simulink model "TosAeb"  (R2021a)
settings: Solver=FixedStepDiscrete  StartTime=0.0  StopTime=inf  FixedStep=0.01  RelTol=1e-3
undefined symbols (not defined in the file or in its folder): ACCModSts, AccSts1, AEB_ObjectMovingOrientation, AEB_ObjectMovingState, CN_BTCActive, CN_CurrentGearR, CN_CurrentGearR_DHT, CN_PTCActive, CN_VDCActive, CN_VSC_EspOff, CN_VSC_FCW_AEB_Error, CN_VSC_FCW_AEB_NoDispay, CN_VSC_FCW_AEB_PerfmDgdtn, CN_VSC_FCW_AEB_TempUnAvlbl, CN_VSC_VehStandstill, CNaccelAvoidMax, CNaccelAvoidMin, CNbrakePedalPress, CNcomfortStrLeftAccelMax, CNcomfortStrLeftAccelMin, CNcomfortStrRightAccelMax, CNcomfortStrRightAccelMin, CNDrvDoorOpen, CNDrvModeEco, CNDrvModeNorm, CNDrvModeOther, CNDrvModeSport, CNDrvSBRreminder, CNNull, CNobjInvalidID, CNPassDoorOpen, CNPassSBRreminder, CNPEBSAccelNegInf, CNPEBSAccelNull, CNPEBSTimeInf, CNPEBSTimeNull, CNSTOPMAX, CNSTOPNULL, CNsubjWidth, CNSwtOFF, CNSwtON, CNTTCMAX, CNTTCNULL, CNVehConfigAEBCycNone, CNVehConfigAEBNone, CNVehConfigAEBPedNone, CNVehConfigFCWNone, CNVehConfigJANone, CollisionSeverity, FALSE, FCWSnvty, JACycObjCalcInfoDefaultParams, JAPedObjCalcInfoDefaultParams, JAVehObjCalcInfoDefaultParams, latCycObjCalcInfoDefaultParams, latPedObjCalcInfoDefaultParams, longObjCalcInfoDefaultParams, ModSts, movingStatus, ObjectType ... (+1112)
libraries: simulink x3031, AEBSFunctionLib x50, Ta_Library x33, AEBSStateMachineLib x23, Mylibrary x12, simulink_extras x7, ObjSelect_lib x3, dspstat3 x2
(part 92 of 102)

== stateflow chart TosAeb/FcwAebJa_Step_sys/ALG_AEBSystem/ALG_AEBController/AEB_JaObjCalcApp/JaCycTgtInfoCalc/TargetSelectCalc/tgtSelectFlow/tgtObjFlow ==
  state TargetSelectFunc [function]
    note: change dy to dx
    (default) -> (junction 63) : {
        | 	tempObjPreSelectCond = ObjPreSelectCondFufilled(index);
        | 	tempFlg = JaCycObjCalcInfo(index).JaCycObjColliInfo.ObjColliFlg;
        | }
    (junction 63) -> (junction 75) [order 1] : [tempObjPreSelectCond== true &&...
        | tempFlg == true &&...
        | abs(SteerWheelAngle_Deg) > P_JaCycCA_SelectStrWheelAngleAbsThres &&...
        | subjVel <= P_JaCycCA_SelectSubjVelThres]
    (junction 63) -> (junction 69) [order 2]
    (junction 75) -> (junction 311)
    (junction 311) -> (junction 312) [order 1] : [ObjPreSelectCondFufilled(index) == false]
    (junction 311) -> (junction 318) [order 2]
    (junction 312) -> (junction 315)
    (junction 318) -> (junction 321) [order 1] : [ObjPreSelectCondFufilled(tgtIndex) == false ||...
        | JaCycObjCalcInfo(index).JaCycObjColliInfo.ObjColliFlg == false]
    (junction 321) -> (junction 323) : {
        | 	tgtIndex = index;
        | }
    (junction 318) -> (junction 128) [order 2] : {
        | 	tgtIsFind = true;
        | 	temp1dx = abs(JaCycObjCalcInfo(index).JaCycObj.dx);
        | 	temp2dx = abs(JaCycObjCalcInfo(tgtIndex).JaCycObj.dx);
        | 	temp1t = JaCycObjCalcInfo(index).JaCycObjColliInfo.tPredictTime;
        | 	temp2t = JaCycObjCalcInfo(tgtIndex).JaCycObjColliInfo.tPredictTime;
        | }
    (junction 128) -> (junction 131) [order 1] : [temp1t < temp2t]
    (junction 128) -> (junction 292) [order 2] : {
        | 	temp = abs(temp1t - temp2t);
        | }
    (junction 131) -> (junction 289) : {tgtIndex = index;}
    (junction 292) -> (junction 294) [order 1] : [temp < 1e-6&&...
        |  temp1dx < temp2dx]
    (junction 292) -> (junction 101) [order 2]
    (junction 294) -> (junction 296) : {tgtIndex = index;}
    (junction 101) -> (junction 69)
    (junction 296) -> (junction 101)
    (junction 289) -> (junction 296)
    (junction 323) -> (junction 289)
    (junction 315) -> (junction 323)
    (junction 69) -> (junction 81)
  (default) -> (junction 111) : {
      | 	tgtIsFind = false;
      | }
  (junction 111) -> (junction 201) [order 1] : [TargetIDK1 ~=  CNobjInvalidID]
  (junction 111) -> (junction 286) [order 2]
  (junction 201) -> (junction 236)
  (junction 236) -> (junction 238) [order 1] : [JACycPrefillModSts == ModSts.ActiveState ||...
      | JACycAEBfullModSts == ModSts.ActiveState ]
  (junction 236) -> (junction 284) [order 2]
  (junction 238) -> (junction 282) : {
      | 	tgtIndex = tgtIndexK1;
      | 	tgtIsFind = true;
      | }
  (junction 284) -> (junction 286)
  (junction 286) -> (junction 112) : {
      | 	tgtIndex = uint8(1);
      | 	index = uint8(1);
      | }
  (junction 112) -> (junction 110) [order 1] : [index <= 32]
  (junction 113) -> (junction 112) : {index = index + 1;}
  (junction 112) -> (junction 114) [order 2]
  (junction 110) -> (junction 113) : {
      | 	TargetSelectFunc();
      | }
  (junction 282) -> (junction 114)
  (junction 114) -> (junction 220)
  (junction 220) -> (junction 222) [order 1] : [tgtIsFind == true]
  (junction 220) -> (junction 223) [order 2] : {
      | 	JaCycTgtObjInfo = JACycObjCalcInfoDefaultParams;
      | }
  (junction 222) -> (junction 221) : {
      | 	JaCycTgtObjInfo = JaCycObjCalcInfo(tgtIndex);
      | }
  (junction 221) -> (junction 223)
  (junction 223) -> (junction 224)
  data: JaCycObjCalcInfo input Bus: BusAEBJaCycObjCalcInfo size=32; index local uint8; JaCycTgtObjInfo output Bus: BusAEBJaCycObjCalcInfo; temp2t local single; temp1t local single; ObjPreSelectCondFufilled input boolean size=32; subjVel input single; SteerWheelAngle_Deg input single; JACycAEBfullModSts input Enum: ModSts; JACycPrefillModSts input Enum: ModSts; TargetIDK1 input uint16; tgtIndex output uint8; tgtIndexK1 input uint8; tgtIsFind local; JACycObjCalcInfoDefaultParams parameter Bus: BusAEBJaCycObjCalcInfo; CNobjInvalidID parameter single; temp1dx local single; temp2dx local single; tempFlg local; tempObjPreSelectCond local boolean; P_JaCycCA_SelectStrWheelAngleAbsThres input single; P_JaCycCA_SelectSubjVelThres input single

== stateflow chart TosAeb/FcwAebJa_Step_sys/ALG_AEBSystem/ALG_AEBController/AEB_JaObjCalcApp/JaCycTgtInfoCalc/JaCycObjCalcInfoCalc/JaCycObjInfoCalc/ObjCrossFlgCal/CollisionProbCalc/Subsystem/ObjLenthWidthCalc/ObjLengthWidthCalc1 ==
  (default) -> (junction 32)
  (junction 32) -> (junction 34) [order 1] : [ObjectTypeCameraConfirmed == true]
  (junction 32) -> (junction 30) [order 2] : {
      | 	ObjectLength = P_LatPedCA_CollisionRiskProbLengthThres;
      | 	ObjectWidth = P_LatPedCA_CollisionRiskProbWidthThres;
      | }
  (junction 34) -> (junction 36) : {
      | 	ObjectLength = ObjectLengthIn;
      | 	ObjectWidth = ObjectWidthIn;
      | }
  (junction 36) -> (junction 30)
  (junction 30) -> (junction 10)
  (junction 10) -> (junction 12) [order 1] : [(abs(ObjectYawAngle) > 45 &&...
      | abs(ObjectYawAngle) < 135)||...
      | ObjectMovingOrientation == AEB_ObjectMovingOrientation.Crossing]
  (junction 10) -> (junction 16) [order 2]
  (junction 12) -> (junction 14) : {
      |       temp = ObjectLength;
      | 	ObjectLength = ObjectWidth;
      | 	ObjectWidth = temp;
      | }
  (junction 14) -> (junction 16)
  (junction 16) -> (junction 19) : {
      |    ObjectLength = min(ObjectLength,2);
      |    ObjectWidth = min(ObjectWidth,1);
      | }
  data: ObjectYawAngle input; ObjectTypeCameraConfirmed input boolean; ObjectLengthIn input; ObjectWidthIn input; ObjectMovingOrientation input; ObjectLength output single; ObjectWidth output single; P_LatPedCA_CollisionRiskProbLengthThres input; P_LatPedCA_CollisionRiskProbWidthThres input; temp local single

== stateflow chart TosAeb/FcwAebJa_Step_sys/ALG_AEBSystem/ALG_AEBInput/LongObjInfoMap/Subsystem/ObjType_Subsystem/ObjectType ==
  (default) -> (junction 17)
  (junction 17) -> (junction 18) [order 1] : [ObjCar==1]
  (junction 17) -> (junction 19) [order 2]
  (junction 18) -> (junction 23) : {ObjType = 2;}
  (junction 19) -> (junction 20) [order 1] : [ObjTruck ==1]
  (junction 19) -> (junction 26) [order 2]
  (junction 20) -> (junction 22) : {
      | 	ObjType=3;
      | }
  (junction 26) -> (junction 27) [order 1] : [ObjPed==1]
  (junction 26) -> (junction 83) [order 2]
  (junction 27) -> (junction 29) : {
      | 	ObjType=6;
      | }
  (junction 83) -> (junction 85) [order 1] : [ObjMotor==1]
  (junction 83) -> (junction 91) [order 2]
  (junction 85) -> (junction 87) : {ObjType=4;}
  (junction 91) -> (junction 93) [order 1] : [ObjBicycle==1]
  (junction 91) -> (junction 100) [order 2]
  (junction 93) -> (junction 95) : {ObjType=5;}
  (junction 100) -> (junction 102) [order 1] : [ObjGeneral==1]
  (junction 100) -> (junction 21) [order 2] : {ObjType=1;}
  (junction 102) -> (junction 104) : {ObjType=7;}
  (junction 104) -> (junction 21)
  (junction 95) -> (junction 104)
  (junction 87) -> (junction 95)
  (junction 29) -> (junction 87)
  (junction 22) -> (junction 29)
  (junction 23) -> (junction 22)
  (junction 21) -> (junction 25)
  data: ObjCar input; ObjTruck input; ObjPed input; ObjType output uint8; ObjMotor input; ObjBicycle input; ObjGeneral input; Unknown input

== stateflow chart TosAeb/FcwAebJa_Step_sys/ALG_AEBSystem/ALG_AEBInput/LatObjInfoMap/latObjInfoMap/Subsystem/ObjType_Subsystem1/ObjectType ==
  (default) -> (junction 17)
  (junction 17) -> (junction 18) [order 1] : [ObjCar==1]
  (junction 17) -> (junction 19) [order 2]
  (junction 18) -> (junction 23) : {ObjType = 2;}
  (junction 19) -> (junction 20) [order 1] : [ObjTruck ==1]
  (junction 19) -> (junction 26) [order 2]
  (junction 20) -> (junction 22) : {
      | 	ObjType=3;
      | }
  (junction 26) -> (junction 27) [order 1] : [ObjPed==1]
  (junction 26) -> (junction 83) [order 2]
  (junction 27) -> (junction 29) : {
      | 	ObjType=6;
      | }
  (junction 83) -> (junction 85) [order 1] : [ObjMotor==1]
  (junction 83) -> (junction 91) [order 2]
  (junction 85) -> (junction 87) : {ObjType=4;}
  (junction 91) -> (junction 93) [order 1] : [ObjBicycle==1]
  (junction 91) -> (junction 100) [order 2]
  (junction 93) -> (junction 95) : {ObjType=5;}
  (junction 100) -> (junction 102) [order 1] : [ObjGeneral==1]
  (junction 100) -> (junction 21) [order 2] : {ObjType=1;}
  (junction 102) -> (junction 104) : {ObjType=7;}
  (junction 104) -> (junction 21)
  (junction 95) -> (junction 104)
  (junction 87) -> (junction 95)
  (junction 29) -> (junction 87)
  (junction 22) -> (junction 29)
  (junction 23) -> (junction 22)
  (junction 21) -> (junction 25)
  data: ObjCar input; ObjTruck input; ObjPed input; ObjType output uint8; ObjMotor input; ObjBicycle input; ObjGeneral input; Unknown input

== stateflow chart TosAeb/FcwAebJa_Step_sys/ALG_AEBSystem/ALG_AEBInput/LongObjInfoMap/Subsystem1/ObjType_Subsystem1/ObjectType ==
  (default) -> (junction 17)
  (junction 17) -> (junction 18) [order 1] : [ObjCar==1]
  (junction 17) -> (junction 19) [order 2]
  (junction 18) -> (junction 23) : {ObjType = 2;}
  (junction 19) -> (junction 20) [order 1] : [ObjTruck ==1]
  (junction 19) -> (junction 26) [order 2]
  (junction 20) -> (junction 22) : {
      | 	ObjType=3;
      | }
  (junction 26) -> (junction 27) [order 1] : [ObjPed==1]
  (junction 26) -> (junction 83) [order 2]
  (junction 27) -> (junction 29) : {
      | 	ObjType=6;
      | }
  (junction 83) -> (junction 85) [order 1] : [ObjMotor==1]
  (junction 83) -> (junction 91) [order 2]
  (junction 85) -> (junction 87) : {ObjType=4;}
  (junction 91) -> (junction 93) [order 1] : [ObjBicycle==1]
  (junction 91) -> (junction 100) [order 2]
  (junction 93) -> (junction 95) : {ObjType=5;}
  (junction 100) -> (junction 102) [order 1] : [ObjGeneral==1]
  (junction 100) -> (junction 21) [order 2] : {ObjType=1;}
  (junction 102) -> (junction 104) : {ObjType=7;}
  (junction 104) -> (junction 21)
  (junction 95) -> (junction 104)
  (junction 87) -> (junction 95)
  (junction 29) -> (junction 87)
  (junction 22) -> (junction 29)
  (junction 23) -> (junction 22)
  (junction 21) -> (junction 25)
  data: ObjCar input; ObjTruck input; ObjPed input; ObjType output uint8; ObjMotor input; ObjBicycle input; ObjGeneral input; Unknown input

== stateflow chart TosAeb/FcwAebJa_Step_sys/ALG_AEBSystem/ALG_AEBInput/LatObjInfoMap/latObjInfoMap/Subsystem1/ObjType_Subsystem1/ObjectType ==
  (default) -> (junction 17)
  (junction 17) -> (junction 18) [order 1] : [ObjCar==1]
  (junction 17) -> (junction 19) [order 2]
  (junction 18) -> (junction 23) : {ObjType = 2;}
  (junction 19) -> (junction 20) [order 1] : [ObjTruck ==1]
  (junction 19) -> (junction 26) [order 2]
  (junction 20) -> (junction 22) : {
      | 	ObjType=3;
      | }
  (junction 26) -> (junction 27) [order 1] : [ObjPed==1]
  (junction 26) -> (junction 83) [order 2]
  (junction 27) -> (junction 29) : {
      | 	ObjType=6;
      | }
  (junction 83) -> (junction 85) [order 1] : [ObjMotor==1]
  (junction 83) -> (junction 91) [order 2]
  (junction 85) -> (junction 87) : {ObjType=4;}
  (junction 91) -> (junction 93) [order 1] : [ObjBicycle==1]
  (junction 91) -> (junction 100) [order 2]
  (junction 93) -> (junction 95) : {ObjType=5;}
  (junction 100) -> (junction 102) [order 1] : [ObjGeneral==1]
  (junction 100) -> (junction 21) [order 2] : {ObjType=1;}
  (junction 102) -> (junction 104) : {ObjType=7;}
  (junction 104) -> (junction 21)
  (junction 95) -> (junction 104)
  (junction 87) -> (junction 95)
  (junction 29) -> (junction 87)
  (junction 22) -> (junction 29)
  (junction 23) -> (junction 22)
  (junction 21) -> (junction 25)
  data: ObjCar input; ObjTruck input; ObjPed input; ObjType output uint8; ObjMotor input; ObjBicycle input; ObjGeneral input; Unknown input

== stateflow chart TosAeb/FcwAebJa_Step_sys/ALG_AEBSystem/ALG_AEBController/AEB_LatObjInfoCalcApp/LatPedObjCalc/Subsystem/latPedObjInfoCalc/ObjCrossFlgCal/PedPreCollisionCalcFunc/ObjLenthWidthCalc/ObjLengthWidthCalc ==
  (default) -> (junction 32)
  (junction 32) -> (junction 34) [order 1] : [ObjectTypeCameraConfirmed == true]
  (junction 32) -> (junction 30) [order 2] : {
      | 	ObjectLength = P_LatPedCA_PreCollisionPedLengthThres;
      | 	ObjectWidth = P_LatPedCA_PreCollisionPedWidthThres;
      | }
  (junction 34) -> (junction 36) : {
      | 	ObjectLength = ObjectLengthIn;
      | 	ObjectWidth = ObjectWidthIn;
      | }
  (junction 36) -> (junction 30)
  (junction 30) -> (junction 10)
  (junction 10) -> (junction 12) [order 1] : [(abs(ObjectYawAngle) > 45 &&...
      | abs(ObjectYawAngle) < 135)||...
      | ObjectMovingOrientation == AEB_ObjectMovingOrientation.Crossing]
  (junction 10) -> (junction 16) [order 2]
  (junction 12) -> (junction 14) : {
      |       temp = ObjectLength;
      | 	ObjectLength = ObjectWidth;
      | 	ObjectWidth = temp;
      | }
  (junction 14) -> (junction 16)
  (junction 16) -> (junction 19) : {
      |    ObjectLength = min(ObjectLength,1.5);
      |    ObjectWidth = min(ObjectWidth,1.5);
      | }
  data: ObjectYawAngle input; ObjectMovingOrientation input; ObjectTypeCameraConfirmed input; ObjectLengthIn input; ObjectWidthIn input; ObjectLength output single; ObjectWidth output single; P_LatPedCA_PreCollisionPedLengthThres input; P_LatPedCA_PreCollisionPedWidthThres input; temp local single

== stateflow chart TosAeb/FcwAebJa_Step_sys/ALG_AEBSystem/ALG_AEBController/AEB_LatObjInfoCalcApp/LatPedObjCalc/Subsystem/latPedObjInfoCalc/ObjCrossFlgCal/PedPreCollisionCalcFunc/PreCrossFlgCalcFlow ==
  state pedPreCollisionFlg = VectorCrossAlgorithm(x1,y1,x2,y2,m1,n1,m2,n2) [function]
  (default) -> (junction 59) : {
      | 	pedPreCollisionFlg = false;
      | 	i = 0;
      | }
  (junction 59) -> (junction 58) [order 1] : [ i < 4]
  (junction 58) -> (junction 48) : {
      | 	p = (i+1)%%4;
      | 	j = 0;
      | }
  (junction 48) -> (junction 46) [order 1] : [j < 4]
  (junction 46) -> (junction 49) : {
      | 	q = (j+1)%%4;
      | 	pedPreCollisionFlg = VectorCrossAlgorithm(subjDxPre[i],subjDyPre[i],subjDxPre[p],subjDyPre[p],...
      | 	objDxPre[j],objDyPre[j],objDxPre[q],objDyPre[q]);
      | }
  (junction 60) -> (junction 59) : {
      | 	i++;
      | }
  (junction 59) -> (junction 61) [order 2]
  (junction 49) -> (junction 48) [order 2] : {
      | 	j++;
      | }
  (junction 48) -> (junction 60) [order 2]
  (junction 49) -> (junction 64) [order 1] : [pedPreCollisionFlg == true]
  (junction 64) -> (junction 61)
  (junction 61) -> (junction 67)
  data: x1 function_input single; y1 function_input single; x2 function_input single; y2 function_input single; m1 function_input single; n1 function_input single; m2 function_input single; n2 function_input single; pedPreCollisionFlg function_output; subjDxPre input single; subjDyPre input single; objDxPre input single; objDyPre input single; j local single; i local single; p local single; q local single; pedPreCollisionFlg output boolean

== stateflow chart TosAeb/FcwAebJa_Step_sys/ALG_AEBSystem/ALG_AEBController/AEB_LatObjInfoCalcApp/LatPedObjCalc/Subsystem/latPedObjInfoCalc/SensorTgtInfo2AbsTgtInfoTrans/ObjFusQualityCal ==
  state [deltaDxThres,deltaDyThres,deltaVyThres] = SensorVyThres(subjVel) [function]
  (default) -> (junction 9)
  (junction 9) -> (junction 11) [order 1] : [ObjectID ~= CNobjInvalidID &&...
      | ObjectTypeCameraConfirmed == true]
  (junction 9) -> (junction 27) [order 2] : {
      |    ObjFusQuality = false;
      | }
  (junction 11) -> (junction 13)
  (junction 13) -> (junction 15) [order 1] : [FR_ObjectID ~= CNobjInvalidID &&...
      | FC_ObjectID ~= CNobjInvalidID]
  (junction 13) -> (junction 24) [order 2] : {
      |    ObjFusQuality = false;
      | }
  (junction 15) -> (junction 17) : {
      |         temp_Dx = abs(FR_Dx - FC_Dx);
      |         temp_Dy = abs(FR_Dy - FC_Dy);
      |         temp_Vy = abs(FR_Vy - FC_Vy);
      |         [deltaDxThres,deltaDyThres,deltaVyThres] = SensorVyThres(subjVel);
      | }
  (junction 17) -> (junction 19) [order 1] : [(abs(FR_Vy) < P_AEB_LatPedObjAbs_SensorFR_VyThres&&...
      | temp_Vy > deltaVyThres &&...
      | abs(dy) > P_AEB_LatPedObjAbs_DyThres4deltaVy)||...
      | temp_Dx > deltaDxThres||...
      | temp_Dy > deltaDyThres ]
  (junction 17) -> (junction 33) [order 2] : {
      |    ObjFusQuality = true;
      | }
  (junction 19) -> (junction 21) : {
      |    ObjFusQuality = false;
      | }
  (junction 24) -> (junction 27)
  (junction 33) -> (junction 24)
  (junction 21) -> (junction 33)
  (junction 27) -> (junction 30)
  data: deltaDxThres function_output single; deltaDyThres function_output single; subjVel function_input; deltaVyThres function_output single; ObjectID input size=1; ObjectTypeCameraConfirmed input size=1; dy input; FR_ObjectID input size=1; FR_Dx input; FR_Dy input; FR_Vy input; FC_ObjectID input size=1; FC_Dx input; FC_Dy input; CNobjInvalidID parameter size=1; ObjFusQuality output size=1; deltaVyThres local single size=1 =single(0); FC_Vy input; P_AEB_LatPedObjAbs_DyThres4deltaVy input; temp_Vy local single; P_AEB_LatPedObjAbs_SensorFR_VyThres input; subjVel input size=1; deltaDxThres local single =single(256); temp_Dx local single; deltaDyThres local single =single(256); temp_Dy local single

== stateflow chart TosAeb/FcwAebJa_Step_sys/ALG_AEBSystem/ALG_AEBInput/JaObjInfoMap/JaObjInfoMap/Subsystem/ObjType_Subsystem1/ObjectType ==
  (default) -> (junction 17)
  (junction 17) -> (junction 18) [order 1] : [ObjCar==1]
  (junction 17) -> (junction 19) [order 2]
  (junction 18) -> (junction 23) : {ObjType = 2;}
  (junction 19) -> (junction 20) [order 1] : [ObjTruck ==1]
  (junction 19) -> (junction 26) [order 2]
  (junction 20) -> (junction 22) : {
      | 	ObjType=3;
      | }
  (junction 26) -> (junction 27) [order 1] : [ObjPed==1]
  (junction 26) -> (junction 83) [order 2]
  (junction 27) -> (junction 29) : {
      | 	ObjType=6;
      | }
  (junction 83) -> (junction 85) [order 1] : [ObjMotor==1]
  (junction 83) -> (junction 91) [order 2]
  (junction 85) -> (junction 87) : {ObjType=4;}
  (junction 91) -> (junction 93) [order 1] : [ObjBicycle==1]
  (junction 91) -> (junction 100) [order 2]
  (junction 93) -> (junction 95) : {ObjType=5;}
  (junction 100) -> (junction 102) [order 1] : [ObjGeneral==1]
  (junction 100) -> (junction 21) [order 2] : {ObjType=1;}
  (junction 102) -> (junction 104) : {ObjType=7;}
  (junction 104) -> (junction 21)
  (junction 95) -> (junction 104)
  (junction 87) -> (junction 95)
  (junction 29) -> (junction 87)
  (junction 22) -> (junction 29)
  (junction 23) -> (junction 22)
  (junction 21) -> (junction 25)
  data: ObjCar input; ObjTruck input; ObjPed input; ObjType output uint8; ObjMotor input; ObjBicycle input; ObjGeneral input; Unknown input

== stateflow chart TosAeb/FcwAebJa_Step_sys/ALG_AEBSystem/ALG_AEBController/AEB_JaObjCalcApp/JaCycTgtInfoCalc/JaCycObjCalcInfoCalc/JaCycObjInfoCalc/SensorTgtInfo2AbsTgtInfoTrans1/ObjFusQualityCal ==
  state deltaDxThres = SensorDxThres(subjVel) [function]
  (default) -> (junction 9)
  (junction 9) -> (junction 11) [order 1] : [ObjectID ~= CNobjInvalidID &&...
      | ObjectTypeCameraConfirmed == true]
  (junction 9) -> (junction 27) [order 2] : {
      |    ObjFusQuality = false;
      | }
  (junction 11) -> (junction 13)
  (junction 13) -> (junction 15) [order 1] : [FR_ObjectID ~= CNobjInvalidID &&...
      | FC_ObjectID ~= CNobjInvalidID]
  (junction 13) -> (junction 24) [order 2] : {
      |    ObjFusQuality = false;
      | }
  (junction 15) -> (junction 17) : {
      |         temp_Dx = abs(FR_Dx - FC_Dx);
      |         deltaDxThres = SensorDxThres(subjVel);
      | }
  (junction 17) -> (junction 19) [order 1] : [temp_Dx < deltaDxThres]
  (junction 17) -> (junction 33) [order 2] : {
      |    ObjFusQuality = false;
      | }
  (junction 19) -> (junction 21) : {
      |    ObjFusQuality = true;
      | }
  (junction 24) -> (junction 27)
  (junction 33) -> (junction 24)
  (junction 21) -> (junction 33)
  (junction 27) -> (junction 30)
  data: subjVel function_input; deltaDxThres function_output single; ObjectID input size=1; ObjectTypeCameraConfirmed input size=1; FC_ObjectID input size=1; FR_ObjectID input size=1; FR_Dx input size=1; FC_Dx input size=1; CNobjInvalidID parameter size=1; ObjFusQuality output size=1; temp_Dx local single size=1; deltaDxThres local single size=1 =single(256); subjVel input size=1

== stateflow chart TosAeb/FcwAebJa_Step_sys/ALG_AEBSystem/ALG_AEBInput/JaObjInfoMap/JaObjInfoMap/Subsystem1/ObjType_Subsystem1/ObjectType ==
  (default) -> (junction 17)
  (junction 17) -> (junction 18) [order 1] : [ObjCar==1]
  (junction 17) -> (junction 19) [order 2]
  (junction 18) -> (junction 23) : {ObjType = 2;}
  (junction 19) -> (junction 20) [order 1] : [ObjTruck ==1]
  (junction 19) -> (junction 26) [order 2]
  (junction 20) -> (junction 22) : {
      | 	ObjType=3;
      | }
  (junction 26) -> (junction 27) [order 1] : [ObjPed==1]
  (junction 26) -> (junction 83) [order 2]
  (junction 27) -> (junction 29) : {
      | 	ObjType=6;
      | }
  (junction 83) -> (junction 85) [order 1] : [ObjMotor==1]
  (junction 83) -> (junction 91) [order 2]
  (junction 85) -> (junction 87) : {ObjType=4;}
  (junction 91) -> (junction 93) [order 1] : [ObjBicycle==1]
  (junction 91) -> (junction 100) [order 2]
  (junction 93) -> (junction 95) : {ObjType=5;}
  (junction 100) -> (junction 102) [order 1] : [ObjGeneral==1]
  (junction 100) -> (junction 21) [order 2] : {ObjType=1;}
  (junction 102) -> (junction 104) : {ObjType=7;}
  (junction 104) -> (junction 21)
  (junction 95) -> (junction 104)
  (junction 87) -> (junction 95)
  (junction 29) -> (junction 87)
  (junction 22) -> (junction 29)
  (junction 23) -> (junction 22)
  (junction 21) -> (junction 25)
  data: ObjCar input; ObjTruck input; ObjPed input; ObjType output uint8; ObjMotor input; ObjBicycle input; ObjGeneral input; Unknown input

== stateflow chart TosAeb/FcwAebJa_Step_sys/ALG_AEBSystem/ALG_AEBInput/LatObjInfoMap/latObjInfoMap/Subsystem/MovingStatus_Subsystem/MovingStatusSwitch ==
  (default) -> (junction 83)
  (junction 83) -> (junction 85) [order 1] : [Input_Moving==1]
  (junction 83) -> (junction 91) [order 2]
  (junction 85) -> (junction 87) : {MovingStatus=3;}
  (junction 91) -> (junction 93) [order 1] : [Input_Stopped==1]
  (junction 91) -> (junction 100) [order 2]
  (junction 93) -> (junction 95) : {MovingStatus=2;}
  (junction 100) -> (junction 102) [order 1] : [Input_Stationary==1]
  (junction 100) -> (junction 21) [order 2] : {MovingStatus=0;}
  (junction 102) -> (junction 104) : {MovingStatus=1;}
  (junction 104) -> (junction 21)
  (junction 95) -> (junction 104)
  (junction 87) -> (junction 95)
  (junction 21) -> (junction 25)
  data: Input_Unknown input; Input_Stationary input; Input_Stopped input; MovingStatus output uint8; Input_Moving input

== stateflow chart TosAeb/FcwAebJa_Step_sys/ALG_AEBSystem/ALG_AEBInput/LatObjInfoMap/latObjInfoMap/Subsystem1/MovingStatus_Subsystem/MovingStatusSwitch ==
  (default) -> (junction 83)
  (junction 83) -> (junction 85) [order 1] : [Input_Moving==1]
  (junction 83) -> (junction 91) [order 2]
  (junction 85) -> (junction 87) : {MovingStatus=3;}
  (junction 91) -> (junction 93) [order 1] : [Input_Stopped==1]
  (junction 91) -> (junction 100) [order 2]
  (junction 93) -> (junction 95) : {MovingStatus=2;}
  (junction 100) -> (junction 102) [order 1] : [Input_Stationary==1]
  (junction 100) -> (junction 21) [order 2] : {MovingStatus=0;}
  (junction 102) -> (junction 104) : {MovingStatus=1;}
  (junction 104) -> (junction 21)
  (junction 95) -> (junction 104)
  (junction 87) -> (junction 95)
  (junction 21) -> (junction 25)
  data: Input_Unknown input; Input_Stationary input; Input_Stopped input; MovingStatus output uint8; Input_Moving input

== stateflow chart TosAeb/FcwAebJa_Step_sys/ALG_AEBSystem/ALG_AEBInput/JaObjInfoMap/JaObjInfoMap/Subsystem/MovingStatus_Subsystem/MovingStatusSwitch ==
  (default) -> (junction 83)
  (junction 83) -> (junction 85) [order 1] : [Input_Moving==1]
  (junction 83) -> (junction 91) [order 2]
  (junction 85) -> (junction 87) : {MovingStatus=3;}
  (junction 91) -> (junction 93) [order 1] : [Input_Stopped==1]
  (junction 91) -> (junction 100) [order 2]
  (junction 93) -> (junction 95) : {MovingStatus=2;}
  (junction 100) -> (junction 102) [order 1] : [Input_Stationary==1]
  (junction 100) -> (junction 21) [order 2] : {MovingStatus=0;}
  (junction 102) -> (junction 104) : {MovingStatus=1;}
  (junction 104) -> (junction 21)
  (junction 95) -> (junction 104)
  (junction 87) -> (junction 95)
  (junction 21) -> (junction 25)
  data: Input_Unknown input; Input_Stationary input; Input_Stopped input; MovingStatus output uint8; Input_Moving input

== stateflow chart TosAeb/FcwAebJa_Step_sys/ALG_AEBSystem/ALG_AEBInput/JaObjInfoMap/JaObjInfoMap/Subsystem1/MovingStatus_Subsystem/MovingStatusSwitch ==
  (default) -> (junction 83)
  (junction 83) -> (junction 85) [order 1] : [Input_Moving==1]
  (junction 83) -> (junction 91) [order 2]
  (junction 85) -> (junction 87) : {MovingStatus=3;}
  (junction 91) -> (junction 93) [order 1] : [Input_Stopped==1]
  (junction 91) -> (junction 100) [order 2]
  (junction 93) -> (junction 95) : {MovingStatus=2;}
  (junction 100) -> (junction 102) [order 1] : [Input_Stationary==1]
  (junction 100) -> (junction 21) [order 2] : {MovingStatus=0;}
  (junction 102) -> (junction 104) : {MovingStatus=1;}
  (junction 104) -> (junction 21)
  (junction 95) -> (junction 104)
  (junction 87) -> (junction 95)
  (junction 21) -> (junction 25)
  data: Input_Unknown input; Input_Stationary input; Input_Stopped input; MovingStatus output uint8; Input_Moving input

== stateflow chart TosAeb/FcwAebJa_Step_sys/ALG_AEBSystem/ALG_AEBInput/LatObjInfoMap/latObjInfoMap/MovingStatus_Subsystem/MovingStatusSwitch ==
  (default) -> (junction 83)
  (junction 83) -> (junction 85) [order 1] : [Input_Moving==1]
  (junction 83) -> (junction 91) [order 2]
  (junction 85) -> (junction 87) : {MovingStatus=3;}
  (junction 91) -> (junction 93) [order 1] : [Input_Stopped==1]
  (junction 91) -> (junction 100) [order 2]
  (junction 93) -> (junction 95) : {MovingStatus=2;}
  (junction 100) -> (junction 102) [order 1] : [Input_Stationary==1]
  (junction 100) -> (junction 21) [order 2] : {MovingStatus=0;}
  (junction 102) -> (junction 104) : {MovingStatus=1;}
  (junction 104) -> (junction 21)
  (junction 95) -> (junction 104)
  (junction 87) -> (junction 95)
  (junction 21) -> (junction 25)
  data: Input_Unknown input; Input_Stationary input; Input_Stopped input; MovingStatus output uint8; Input_Moving input

== stateflow chart TosAeb/FcwAebJa_Step_sys/ALG_AEBSystem/ALG_AEBInput/JaObjInfoMap/JaObjInfoMap/MovingStatus_Subsystem/MovingStatusSwitch ==
  (default) -> (junction 83)
  (junction 83) -> (junction 85) [order 1] : [Input_Moving==1]
  (junction 83) -> (junction 91) [order 2]
  (junction 85) -> (junction 87) : {MovingStatus=3;}
  (junction 91) -> (junction 93) [order 1] : [Input_Stopped==1]
  (junction 91) -> (junction 100) [order 2]
  (junction 93) -> (junction 95) : {MovingStatus=2;}
  (junction 100) -> (junction 102) [order 1] : [Input_Stationary==1]
  (junction 100) -> (junction 21) [order 2] : {MovingStatus=0;}
  (junction 102) -> (junction 104) : {MovingStatus=1;}
  (junction 104) -> (junction 21)
  (junction 95) -> (junction 104)
  (junction 87) -> (junction 95)
  (junction 21) -> (junction 25)
  data: Input_Unknown input; Input_Stationary input; Input_Stopped input; MovingStatus output uint8; Input_Moving input

== matlab function TosAeb/AEB_Objsel/TA_InfoRebuild/TAObjInfoCalc/For Each Subsystem/Info_rebuild/Road_relative/LineInfo_Process/LineInfo_Process_Left/Line_Info ==
  data: seg_num input; seg1_valid input; Valid output; seg1_Start input; seg1_end input; seg1_C0 input; seg1_C1 input; seg1_C2 input; seg1_C3 input; seg2_valid input; seg2_Start input; seg2_end input; seg2_C0 input; seg2_C1 input; seg2_C2 input; seg2_C3 input; seg3_valid input; seg3_Start input; seg3_end input; seg3_C0 input; seg3_C1 input; seg3_C2 input; seg3_C3 input; Line_start output; Line_end output; C0 output; C1 output; C2 output; C3 output
function [Valid,Line_start,Line_end,C0,C1,C2,C3] = fcn(seg_num,seg1_valid,seg1_Start,seg1_end,seg1_C0,seg1_C1,seg1_C2,seg1_C3,...
    seg2_valid,seg2_Start,seg2_end,seg2_C0,seg2_C1,seg2_C2,seg2_C3,...
    seg3_valid,seg3_Start,seg3_end,seg3_C0,seg3_C1,seg3_C2,seg3_C3)
posx = single(zeros(20,1));
posy = single(zeros(20,1));
Valid = false;
Line_start = single(0);
Line_end = single(0);
C0 = single(0);
C1 = single(0);
C2 = single(0);
C3 = single(0);
%确定有效分段数目
if seg_num == uint8(0)

    %只有一段距离有效，输出有效段参数，后续需要增加距离判断拟合
elseif seg_num == uint8(1)
    if seg1_valid == true && seg2_valid ==false && seg3_valid == false
        [Valid,Line_start,Line_end,C0,C1,C2,C3] = func_assign(seg1_valid,seg1_Start,seg1_end,seg1_C0,seg1_C1,seg1_C2,seg1_C3);
    elseif seg2_valid == true && seg1_valid ==false && seg3_valid == false
        [Valid,Line_start,Line_end,C0,C1,C2,C3] = func_assign(seg2_valid,seg2_Start,seg2_end,seg2_C0,seg2_C1,seg2_C2,seg2_C3);
    elseif seg3_valid == true && seg1_valid ==false && seg2_valid == false
        [Valid,Line_start,Line_end,C0,C1,C2,C3] = func_assign(seg3_valid,seg3_Start,seg3_end,seg3_C0,seg3_C1,seg3_C2,seg3_C3);
    else

    end
    %存在两端距离有效，判断是1、2，2、3，或者1、3（当前未考虑C0拼接的问题，需根据后续实车数据查看偏差对功能影响程度）
elseif seg_num == uint8(2)
    %1、2段距离有效
    if seg1_valid == true && seg2_valid == true && seg3_valid == false
        if seg1_Start > seg2_Start     %1、2段存在重叠
            if seg2_Start > single(0)  %1段的有效距离大于零
                seg2_Start = seg1_Start;
            else
                %seg1_Start = seg2_Start;
            end
        else
            seg2_Start  = seg1_Start;
        end
        t_size = func_step(seg1_end,seg2_end);
        for i = 1:20
            t_dx = seg1_end + (i - 1)*t_size;
            posx(i) = t_dx;
            if t_dx <= seg2_Start
                posy(i) = func_takePoint(seg1_C0,seg1_C1,seg1_C2,seg1_C3,t_dx);
            else
                posy(i) = func_takePoint(seg2_C0,seg2_C1,seg2_C2,seg2_C3,t_dx);
            end
        end
        coef = polyfit(posx,posy,3);
        Valid = true;
        Line_start = seg1_end;
        Line_end = seg2_end;
        C0 = coef(4);
        C1 = coef(3);
        C2 = coef(2);
        C3 = coef(1);
        %2、3段距离有效
    elseif seg1_valid == false && seg2_valid == true && seg3_valid == true
        if seg2_Start > single(30)       %(此处阈值的设置最好可以根据车速进行插值设置，后续需要根据实车数据进行处理)
            Valid = false;
            Line_start = single(0);
            Line_end = single(0);
            C0 = single(0);
            C1 = single(0);
            C2 = single(0);
            C3 = single(0);
        else
            if seg2_end < seg3_end
                if seg2_end <= seg3_Start     %2、3段存在重叠
                    seg3_Start = seg2_end;
                end
            else
                seg3_end = seg2_end;
            end
            t_size = func_step(seg2_Start,seg3_end);
            for i = 1:20
                t_dx = seg2_Start + (i - 1)*t_size;
                posx(i) = t_dx;
                if t_dx <= seg3_Start
                    posy(i) = func_takePoint(seg2_C0,seg2_C1,seg2_C2,seg2_C3,t_dx);
                else
                    posy(i) = func_takePoint(seg3_C0,seg3_C1,seg3_C2,seg3_C3,t_dx);
                end
            end
            coef = polyfit(posx,posy,3);
            Valid = true;
            Line_start = seg2_Start;
            Line_end = seg3_end;
            C0 = coef(4);
            C1 = coef(3);
            C2 = coef(2);
            C3 = coef(1);
        end


        %1、3段距离有效
    elseif seg1_valid == true && seg2_valid == false && seg3_valid == true
        if seg3_Start - seg1_Start > single(30)
            seg3_end = seg1_Start;

        else
            seg1_Start = (seg3_Start - seg1_Start)*single(0.5) + seg1_Start;

        end
        t_size = func_step(seg1_end,seg3_end);
        for i = 1:20
            t_dx = seg2_Start + (i - 1)*t_size;
            posx(i) = t_dx;
            if t_dx <= seg1_Start
                posy(i) = func_takePoint(seg1_C0,seg1_C1,seg1_C2,seg1_C3,t_dx);
            else
                posy(i) = func_takePoint(seg3_C0,seg3_C1,seg3_C2,seg3_C3,t_dx);
            end
        end
        coef = polyfit(posx,posy,3);
        Valid = true;
        Line_start = seg1_end;
        Line_end = seg3_end;
        C0 = coef(4);
        C1 = coef(3);
        C2 = coef(2);
        C3 = coef(1);
    end
    %三段有效
else
    if seg1_Start > seg2_Start
        seg2_Start = seg1_Start;
    end
    if seg2_end > seg3_Start
        seg2_end = seg3_Start;
    end
    if seg3_Start >= single(150)
        t_size = func_step(seg1_end,seg2_end);
        for i = 1:20
            t_dx = seg2_Start + (i - 1)*t_size;
            posx(i) = t_dx;
            if t_dx <= seg1_Start
                posy(i) = func_takePoint(seg1_C0,seg1_C1,seg1_C2,seg1_C3,t_dx);
            else
                posy(i) = func_takePoint(seg2_C0,seg2_C1,seg2_C2,seg2_C3,t_dx);

            end
        end
    else
        t_size = func_step(seg1_end,seg3_end);
        for i = 1:20
            t_dx = seg2_Start + (i - 1)*t_size;
            posx(i) = t_dx;
            if t_dx <= seg1_Start
                posy(i) = func_takePoint(seg1_C0,seg1_C1,seg1_C2,seg1_C3,t_dx);
            elseif t_dx > seg1_Start && t_dx <= seg2_end
                posy(i) = func_takePoint(seg2_C0,seg2_C1,seg2_C2,seg2_C3,t_dx);
            else

                posy(i) = func_takePoint(seg3_C0,seg3_C1,seg3_C2,seg3_C3,t_dx);
            end
        end
    end

    coef = polyfit(posx,posy,3);
    Valid = true;
    Line_start = seg1_end;
    Line_end = seg3_end;
    C0 = coef(4);
    C1 = coef(3);
    C2 = coef(2);
    C3 = coef(1);
end
end

%%
%赋值检查函数
function [t_flg,t_start,t_end,t_c0,t_c1,t_c2,t_c3] = func_assign(seg_flg,seg_start,seg_end,seg_c0,seg_c1,seg_c2,seg_c3)
if seg_flg ~= false && seg_start ~= single(0) && seg_end ~= single(0) && seg_c0 ~= single(0) && seg_c1 ~= single(0) && seg_c2 ~= single(0) && seg_c3 ~= single(0)
    t_flg = seg_flg;
    t_start = seg_start;
    t_end = seg_end;
    t_c0 = seg_c0;
    t_c1 = seg_c1;
    t_c2 = seg_c2;
    t_c3 = seg_c3;
else
    t_flg = false;
    t_start = single(0);
    t_end = single(0);
    t_c0 = single(0);
    t_c1 = single(0);
    t_c2 = single(0);
    t_c3 = single(0);
end
end
% 离散取点函数
function y = func_takePoint(a0,a1,a2,a3,x)
y = a0 + a1*x + a2*x*x + a3*x*x*x;
end

% 取值步长的计算
function size = func_step(t1,t2)
if t2 > single(150)
    t2 = single(150);
end
size = (t2 - t1)* single(0.05);
end

== matlab function TosAeb/AEB_Objsel/TA_InfoRebuild/TAObjInfoCalc/For Each Subsystem/Info_rebuild/Road_relative/LineInfo_Process/LineInfo_Process_Right/Line_Info ==
  data: seg_num input; seg1_valid input; Valid output; seg1_Start input; seg1_end input; seg1_C0 input; seg1_C1 input; seg1_C2 input; seg1_C3 input; seg2_valid input; seg2_Start input; seg2_end input; seg2_C0 input; seg2_C1 input; seg2_C2 input; seg2_C3 input; seg3_valid input; seg3_Start input; seg3_end input; seg3_C0 input; seg3_C1 input; seg3_C2 input; seg3_C3 input; Line_start output; Line_end output; C0 output; C1 output; C2 output; C3 output
function [Valid,Line_start,Line_end,C0,C1,C2,C3] = fcn(seg_num,seg1_valid,seg1_Start,seg1_end,seg1_C0,seg1_C1,seg1_C2,seg1_C3,...
    seg2_valid,seg2_Start,seg2_end,seg2_C0,seg2_C1,seg2_C2,seg2_C3,...
    seg3_valid,seg3_Start,seg3_end,seg3_C0,seg3_C1,seg3_C2,seg3_C3)
posx = single(zeros(20,1));
posy = single(zeros(20,1));
Valid = false;
Line_start = single(0);
Line_end = single(0);
C0 = single(0);
C1 = single(0);
C2 = single(0);
C3 = single(0);
%确定有效分段数目
if seg_num == uint8(0)

    %只有一段距离有效，输出有效段参数，后续需要增加距离判断拟合
elseif seg_num == uint8(1)
    if seg1_valid == true && seg2_valid ==false && seg3_valid == false
        [Valid,Line_start,Line_end,C0,C1,C2,C3] = func_assign(seg1_valid,seg1_Start,seg1_end,seg1_C0,seg1_C1,seg1_C2,seg1_C3);
    elseif seg2_valid == true && seg1_valid ==false && seg3_valid == false
        [Valid,Line_start,Line_end,C0,C1,C2,C3] = func_assign(seg2_valid,seg2_Start,seg2_end,seg2_C0,seg2_C1,seg2_C2,seg2_C3);
    elseif seg3_valid == true && seg1_valid ==false && seg2_valid == false
        [Valid,Line_start,Line_end,C0,C1,C2,C3] = func_assign(seg3_valid,seg3_Start,seg3_end,seg3_C0,seg3_C1,seg3_C2,seg3_C3);
    else

    end
    %存在两端距离有效，判断是1、2，2、3，或者1、3（当前未考虑C0拼接的问题，需根据后续实车数据查看偏差对功能影响程度）
elseif seg_num == uint8(2)
    %1、2段距离有效
    if seg1_valid == true && seg2_valid == true && seg3_valid == false
        if seg1_Start > seg2_Start     %1、2段存在重叠
            if seg2_Start > single(0)  %1段的有效距离大于零
                seg2_Start = seg1_Start;
            else
                %seg1_Start = seg2_Start;
            end
        else
            seg2_Start  = seg1_Start;
        end
        t_size = func_step(seg1_end,seg2_end);
        for i = 1:20
            t_dx = seg1_end + (i - 1)*t_size;
            posx(i) = t_dx;
            if t_dx <= seg2_Start
                posy(i) = func_takePoint(seg1_C0,seg1_C1,seg1_C2,seg1_C3,t_dx);
            else
                posy(i) = func_takePoint(seg2_C0,seg2_C1,seg2_C2,seg2_C3,t_dx);
            end
        end
        coef = polyfit(posx,posy,3);
        Valid = true;
        Line_start = seg1_end;
        Line_end = seg2_end;
        C0 = coef(4);
        C1 = coef(3);
        C2 = coef(2);
        C3 = coef(1);
        %2、3段距离有效
    elseif seg1_valid == false && seg2_valid == true && seg3_valid == true
        if seg2_Start > single(30)       %(此处阈值的设置最好可以根据车速进行插值设置，后续需要根据实车数据进行处理)
            Valid = false;
            Line_start = single(0);
            Line_end = single(0);
            C0 = single(0);
            C1 = single(0);
            C2 = single(0);
            C3 = single(0);
        else
            if seg2_end < seg3_end
                if seg2_end <= seg3_Start     %2、3段存在重叠
                    seg3_Start = seg2_end;
                end
            else
                seg3_end = seg2_end;
            end
            t_size = func_step(seg2_Start,seg3_end);
            for i = 1:20
                t_dx = seg2_Start + (i - 1)*t_size;
                posx(i) = t_dx;
                if t_dx <= seg3_Start
                    posy(i) = func_takePoint(seg2_C0,seg2_C1,seg2_C2,seg2_C3,t_dx);
                else
                    posy(i) = func_takePoint(seg3_C0,seg3_C1,seg3_C2,seg3_C3,t_dx);
                end
            end
            coef = polyfit(posx,posy,3);
            Valid = true;
            Line_start = seg2_Start;
            Line_end = seg3_end;
            C0 = coef(4);
            C1 = coef(3);
            C2 = coef(2);
            C3 = coef(1);
        end


        %1、3段距离有效
    elseif seg1_valid == true && seg2_valid == false && seg3_valid == true
        if seg3_Start - seg1_Start > single(30)
            seg3_end = seg1_Start;

        else
            seg1_Start = (seg3_Start - seg1_Start)*single(0.5) + seg1_Start;

        end
        t_size = func_step(seg1_end,seg3_end);
        for i = 1:20
            t_dx = seg2_Start + (i - 1)*t_size;
            posx(i) = t_dx;
            if t_dx <= seg1_Start
                posy(i) = func_takePoint(seg1_C0,seg1_C1,seg1_C2,seg1_C3,t_dx);
            else
                posy(i) = func_takePoint(seg3_C0,seg3_C1,seg3_C2,seg3_C3,t_dx);
            end
        end
        coef = polyfit(posx,posy,3);
        Valid = true;
        Line_start = seg1_end;
        Line_end = seg3_end;
        C0 = coef(4);
        C1 = coef(3);
        C2 = coef(2);
        C3 = coef(1);
    end
    %三段有效
else
    if seg1_Start > seg2_Start
        seg2_Start = seg1_Start;
    end
    if seg2_end > seg3_Start
        seg2_end = seg3_Start;
    end
    if seg3_Start >= single(150)
        t_size = func_step(seg1_end,seg2_end);
        for i = 1:20
            t_dx = seg2_Start + (i - 1)*t_size;
            posx(i) = t_dx;
            if t_dx <= seg1_Start
                posy(i) = func_takePoint(seg1_C0,seg1_C1,seg1_C2,seg1_C3,t_dx);
            else
                posy(i) = func_takePoint(seg2_C0,seg2_C1,seg2_C2,seg2_C3,t_dx);

            end
        end
    else
        t_size = func_step(seg1_end,seg3_end);
        for i = 1:20
            t_dx = seg2_Start + (i - 1)*t_size;
            posx(i) = t_dx;
            if t_dx <= seg1_Start
                posy(i) = func_takePoint(seg1_C0,seg1_C1,seg1_C2,seg1_C3,t_dx);
            elseif t_dx > seg1_Start && t_dx <= seg2_end
                posy(i) = func_takePoint(seg2_C0,seg2_C1,seg2_C2,seg2_C3,t_dx);
            else

                posy(i) = func_takePoint(seg3_C0,seg3_C1,seg3_C2,seg3_C3,t_dx);
            end
        end
    end

    coef = polyfit(posx,posy,3);
    Valid = true;
    Line_start = seg1_end;
    Line_end = seg3_end;
    C0 = coef(4);
    C1 = coef(3);
    C2 = coef(2);
    C3 = coef(1);
end
end

%%
%赋值检查函数
function [t_flg,t_start,t_end,t_c0,t_c1,t_c2,t_c3] = func_assign(seg_flg,seg_start,seg_end,seg_c0,seg_c1,seg_c2,seg_c3)
if seg_flg ~= false && seg_start ~= single(0) && seg_end ~= single(0) && seg_c0 ~= single(0) && seg_c1 ~= single(0) && seg_c2 ~= single(0) && seg_c3 ~= single(0)
    t_flg = seg_flg;
    t_start = seg_start;
    t_end = seg_end;
    t_c0 = seg_c0;
    t_c1 = seg_c1;
    t_c2 = seg_c2;
    t_c3 = seg_c3;
else
    t_flg = false;
    t_start = single(0);
    t_end = single(0);
    t_c0 = single(0);
    t_c1 = single(0);
    t_c2 = single(0);
    t_c3 = single(0);
end
end
% 离散取点函数
function y = func_takePoint(a0,a1,a2,a3,x)
y = a0 + a1*x + a2*x*x + a3*x*x*x;
end

% 取值步长的计算
function size = func_step(t1,t2)
if t2 > single(150)
    t2 = single(150);
end
size = (t2 - t1)* single(0.05);
end

== matlab function TosAeb/AEB_Objsel/TA_InfoRebuild/TAObjInfoCalc/For Each Subsystem/Info_rebuild/Road_relative/RebuildInfo_Process/If Action Subsystem1/MATLAB Function ==
  data: dx input; objLatDist2LaneCenter output; dy input; left_valid input; left_C0 input; left_C1 input; left_C2 input; left_C3 input; right_valid input; right_C0 input; right_C1 input; right_C2 input; right_C3 input; objLatDist2EgoCenter output; objPosition output
function [objLatDist2LaneCenter,objLatDist2EgoCenter,objPosition] = fcn(dx,dy,left_valid,left_C0,left_C1,left_C2,left_C3,right_valid,right_C0,right_C1,right_C2,right_C3)
if left_valid == true && right_valid == true
    C0 = single((left_C0 + right_C0)*0.5);
    C1 = single((left_C1 + right_C1)*0.5);
    C2 = single((left_C2 + right_C2)*0.5);
    C3 = single((left_C3 + right_C3)*0.5);
    t_y = C3*(dx*dx*dx) + C2*(dx*dx) + C1*dx +C0;
    objLatDist2LaneCenter = dy - t_y;
    objLatDist2EgoCenter = dy;
    objPosition = func_Calculate(dx,dy,t_y);
elseif left_valid == true && right_valid == false
    %C0 = single(left_C0);
    C1 = single(left_C1);
    C2 = single(left_C2);
    C3 = single(left_C3);
    t_y = C3*(dx*dx*dx) + C2*(dx*dx) + C1*dx;
    objLatDist2LaneCenter = dy - t_y;
    objLatDist2EgoCenter = dy;
    objPosition = func_Calculate(dx,dy,t_y);
elseif left_valid == false && right_valid == true
    %C0 = single(left_C0);
    C1 = single(right_C1);
    C2 = single(right_C2);
    C3 = single(right_C3);
    t_y = C3*(dx*dx*dx) + C2*(dx*dx) + C1*dx;
    objLatDist2LaneCenter = dy - t_y;
    objLatDist2EgoCenter = dy;
    objPosition = func_Calculate(dx,dy,t_y);
else
    t_y =single(0);
    objLatDist2LaneCenter = dy - t_y;
    objLatDist2EgoCenter = dy;
    objPosition = func_Calculate(dx,dy,t_y);
end
end

function t_objposition = func_Calculate(x,y,ty)
if x >  single(2.5)%前方
    if y > ty - single(1.75)&& y < ty + single(1.75)
        t_objposition = uint8(10);%前方
    elseif y <ty - single(1.75)
        t_objposition = uint8(80);%右前
    else
        t_objposition = uint8(20);%左前
    end
elseif  x < single(2.5)&& x >single(-2.5) %在车辆左右两侧
    if abs(ty) < single(1.25)
        t_objposition = uint8(0);
    elseif ty >single(1.25)
        t_objposition = uint8(30);%左侧
    else
        t_objposition = uint8(70);%右侧
    end
else
    if y > ty - single(1.75)&& y < ty + single(1.75)
        t_objposition = uint8(50);%后方
    elseif y <ty - single(1.75)
        t_objposition = uint8(60);%右后
    else
        t_objposition = uint8(40);%左后
    end
end
end

== stateflow chart TosAeb/AEB_Objsel/TA_Processor/AEB_ObjSelect/TargetInfoCheck/Data_precess/TA_EffectCalculate/Obj_FilterFlgCalculate/JA_ObjFilter/CollisionProbCalc/Subsystem/PreCrossFlgCalcFlow ==
  state preCrossFlg = VectorCrossAlgorithm(x1,y1,x2,y2,m1,n1,m2,n2) [function]
  (default) -> (junction 59) : {
      | 	preCrossFlg = false;
      | 	i = 0;
      | }
  (junction 59) -> (junction 58) [order 1] : [ i < 4]
  (junction 58) -> (junction 48) : {
      | 	p = (i+1)%%4;
      | 	j = 0;
      | }
  (junction 48) -> (junction 46) [order 1] : [j < 4]
  (junction 46) -> (junction 49) : {
      | 	q = (j+1)%%4;
      | 	preCrossFlg = VectorCrossAlgorithm(subjDxPre[i],subjDyPre[i],subjDxPre[p],subjDyPre[p],...
      | 	objDxPre[j],objDyPre[j],objDxPre[q],objDyPre[q]);
      | }
  (junction 60) -> (junction 59) : {
      | 	i++;
      | }
  (junction 59) -> (junction 61) [order 2]
  (junction 49) -> (junction 48) [order 2] : {
      | 	j++;
      | }
  (junction 48) -> (junction 60) [order 2]
  (junction 49) -> (junction 64) [order 1] : [preCrossFlg == true]
  (junction 64) -> (junction 61)
  (junction 61) -> (junction 67)
  data: x1 function_input single; y1 function_input single; x2 function_input single; y2 function_input single; m1 function_input single; n1 function_input single; m2 function_input single; n2 function_input single; preCrossFlg function_output; subjDxPre input single; subjDyPre input single; objDxPre input single; objDyPre input single; j local single; i local single; p local single; q local single; preCrossFlg output boolean

== matlab function TosAeb/AEB_Objsel/TA_Processor/AEB_ObjSelect/TargetInfoCheck/Data_precess/TA_EffectCalculate/Obj_FilterFlgCalculate/Lat_ObjFilter/Lat_Objelse/Lat_ObjPre/Subsystem/LatDis_Cal/L2R/L2R_Calculate ==
  data: dy input; Valid_Flg output; Width_Large input; dx input; ax input; ay input; vx input; vy input; TTI output
function [Valid_Flg,TTI] = fcn(dy,Width_Large,dx,ax,ay,vx,vy)
%Valid_Flg = false;
%TTI = single(100);
distance_dx = dx;
% 目标处于车辆右侧，无重叠度，向右行驶；
if dy <= single(0) && abs(dy) > Width_Large && vy < single(0)
    Valid_Flg = false;
    TTI = single(100);
    % 目标处于车辆右侧，存在重叠，向右行驶
elseif dy <= single(0) && abs(dy) <= Width_Large && vy < single(0)
    distance_y1 = abs(dy) - Width_Large;
    if distance_y1 * vy >single(0)
        distance_y1 = single(-1)*distance_y1;
    else
    end
    time_1 = solution(single(0.5)*ay,vy,distance_y1);
    distance_x1 = abs(vx)*time_1 + single(0.5)*ax*(time_1)^2;
    time = solution(single(0.5)*ax,vx,distance_dx);
    if distance_x1 <= single(0) || time > single(10) || time_1 >single(10)
        Valid_Flg = false;
        TTI = single(100);
    else
        Valid_Flg = true;
        TTI = time;

    end
    % 目标处于车辆左侧，存在重叠，向右行驶
elseif dy >= single(0) && abs(dy) <= Width_Large && vy < single(0)
    distance_y1 = dy - Width_Large;
    if distance_y1 * vy >single(0)
        distance_y1 = single(-1)*distance_y1;
    else
    end
    time_1 = solution(single(0.5)*ay,vy,distance_y1);
    distance_x1 = abs(vx)*time_1 + single(0.5)*ax*(time_1)^2;
    time = solution(single(0.5)*ax,vx,distance_dx);
    if distance_x1 <= single(0) || time > single(10) || time_1 >single(10)
        Valid_Flg = false;
        TTI = single(100);
    else

        Valid_Flg = true;
        TTI = time;


    end
    % 目标处于车辆左侧，不存在重叠，向右行驶
elseif dy > single(0) && abs(dy) > Width_Large && vy < single(0)
    distance_y1 = dy - Width_Large;
    distance_y2 = dy + Width_Large;
    time_1 = solution(single(0.5)*ay,vy,distance_y1);
    time_2 = solution(single(0.5)*ay,vy,distance_y2);
    distance_x1 = abs(vx)*time_1 + single(0.5)*ax*(time_1)^2;
    distance_x2 = abs(vx)*time_2 + single(0.5)*ax*(time_2)^2;
    time = solution(single(0.5)*ax,vx,distance_dx);
    if distance_x1 <= single(0) || distance_x2 <= single(0) || time > single(10) || time_1 >single(10)||time_2 >single(10)
        Valid_Flg = false;
        TTI = single(100);
    else

        Valid_Flg = true;
        TTI = time;

    end
else
    Valid_Flg = false;
    TTI = single(100);
end
end
%% 一元二次方程求解
function result = solution(a,b,c)
d = single(1) *c;
delta = single(b*b - 4*a*d);
if abs(a) <= single(0.01)  && b~= single(0)
    result =  abs(single(c/b));
else
    if delta > single(0)
        r1 = single(((-b) - sqrt(delta))/(2*a));
        r2 = single(((-b) + sqrt(delta))/(2*a));
        if r1 > single(0) && r2 >single(0)
            result = min(r1,r2);
        elseif r1 > single(0) && r2 <= single(0)
            result = r1;
        elseif r1 <= single(0) && r2 > single(0)
            result = r2;
        else
            result = single(100);
        end
    elseif delta == single(0)
        r1 = single((-b)/(2*a));
        r2 = r1;
        if r2 > single(0)
            result = r2;
        else
            result = single(100);
        end
    else
        result = single(100);
    end
end
end
%%
